simulink model "fftwbr_core"  (Simulink 7.9)
settings: Solver=ode45  StartTime=0.0  StopTime=10.0  RelTol=1e-3
libraries: xbsIndex_r4 x15792, casper_library_misc x464, casper_library_delays x57, xbsReplacements_r4 x8, casper_library_ffts_twiddle x4
(part 135 of 233)

== system fftwbr_core/fft_wideband_real1/fft_direct/butterfly4_4/twiddle_general_dsp48e/coeff_gen ==
  rst : Inport
  Counter : xbsIndex_r4/Counter  cnt_type=Free Running  cnt_to=Inf  operation=Up  start_count=0  cnt_by_val=1  arith_type=Unsigned  n_bits=11  bin_pt=0  load_pin=off  rst=on  en=off  explicit_period=on  period=1  dbl_ovrd=off  use_behavioral_HDL=off  implementation=Fabric  block_type=counter
  ROM : xbsIndex_r4/ROM  depth=2048  initVector="[0.9238739013671875                     0.9238128662109375                      0.923736572265625                     0.9236602783203125                         0.923583984375                     0.9235076904296875                      0.923431396484375                      0.923370361328125                     0.9232940673828125                        0.9232177734375                     0.9231414794921875                      0.923065185546875                      0.923004150390625                     0.9229278564453125                           0.9228515625                     0.9227752685546875                      0.922698974609375                     0.9226226806640625                       0.92254638671875                        0.9224853515625                     0.9224090576171875                      0.922332763671875                     0.9222564697265625                       0.92218017578125                     0.9221038818359375                      0.922027587890625                      0.921966552734375                     0.9218902587890625                       0.92181396484375                     0.9217376708984375                      0.921661376953125                     0.9215850830078125                        0.9215087890625                     0.9214324951171875                     0.9213714599609375                      0.921295166015625                     0.9212188720703125                         0.921142578125                     0.9210662841796875                      0.920989990234375                     0.9209136962890625                       0.92083740234375                     0.9207611083984375                     0.9207000732421875                      0.920623779296875                     0.9205474853515625                       0.92047119140625                     0.9203948974609375                      0.920318603515625                     0.9202423095703125                         0.920166015625                     0.9200897216796875                      0.920013427734375                     0.9199371337890625                       0.91986083984375                        0.9197998046875                     0.9197235107421875                      0.919647216796875                     0.9195709228515625                       0.91949462890625                     0.9194183349609375                      0.919342041015625                     0.9192657470703125                         0.919189453125                     0.9191131591796875                      0.919036865234375                     0.9189605712890625                       0.91888427734375                     0.9188079833984375                      0.918731689453125                     0.9186553955078125                        0.9185791015625                     0.9185028076171875                      0.918426513671875                     0.9183502197265625                       0.91827392578125                     0.9181976318359375                      0.918121337890625                      0.918060302734375                     0.9179840087890625                       0.91790771484375                     0.9178314208984375                      0.917755126953125                     0.9176788330078125                        0.9176025390625                     0.9175262451171875                      0.917449951171875                     0.9173736572265625                       0.91729736328125                     0.9172210693359375                      0.917144775390625                     0.9170684814453125                           0.9169921875                     0.9169158935546875                      0.916839599609375                         0.916748046875                     0.9166717529296875                      0.916595458984375                     0.9165191650390625                       0.91644287109375                     0.9163665771484375                      0.916290283203125                     0.9162139892578125 ...(+75853 chars)]"  distributed_mem=Block RAM  rst=off  init_reg=0  en=off  latency=2  gui_display_data_type=Fixed-point  arith_type="Signed  (2's comp)"  n_bits=18  bin_pt=16  preci_type=Single  exp_width=8  frac_width=24  dbl_ovrd=off  optimize=Area  use_rpm=on  block_type=sprom
  ROM1 : xbsIndex_r4/ROM  depth=2048  initVector="[-0.3826904296875                    -0.3828582763671875                    -0.3830413818359375                     -0.383209228515625                     -0.383392333984375                     -0.383575439453125                    -0.3837432861328125                    -0.3839263916015625                      -0.38409423828125                         -0.38427734375                      -0.38446044921875                    -0.3846282958984375                    -0.3848114013671875                     -0.384979248046875                     -0.385162353515625                     -0.385345458984375                    -0.3855133056640625                    -0.3856964111328125                       -0.3858642578125                      -0.38604736328125                         -0.38623046875                    -0.3863983154296875                    -0.3865814208984375                     -0.386749267578125                     -0.386932373046875                    -0.3871002197265625                    -0.3872833251953125                    -0.3874664306640625                      -0.38763427734375                       -0.3878173828125                    -0.3879852294921875                    -0.3881683349609375                    -0.3883514404296875                     -0.388519287109375                     -0.388702392578125                    -0.3888702392578125                    -0.3890533447265625                      -0.38922119140625                        -0.389404296875                      -0.38958740234375                    -0.3897552490234375                    -0.3899383544921875                     -0.390106201171875                     -0.390289306640625                    -0.3904571533203125                    -0.3906402587890625                    -0.3908233642578125                       -0.3909912109375                      -0.39117431640625                    -0.3913421630859375                    -0.3915252685546875                     -0.391693115234375                     -0.391876220703125                     -0.392059326171875                    -0.3922271728515625                    -0.3924102783203125                           -0.392578125                      -0.39276123046875                    -0.3929290771484375                    -0.3931121826171875                     -0.393280029296875                     -0.393463134765625                     -0.393646240234375                    -0.3938140869140625                    -0.3939971923828125                       -0.3941650390625                      -0.39434814453125                    -0.3945159912109375                    -0.3946990966796875                     -0.394866943359375                     -0.395050048828125                    -0.3952178955078125                    -0.3954010009765625                    -0.3955841064453125                        -0.395751953125                      -0.39593505859375                    -0.3961029052734375                    -0.3962860107421875                     -0.396453857421875                     -0.396636962890625                    -0.3968048095703125                    -0.3969879150390625                      -0.39715576171875                       -0.3973388671875                    -0.3975067138671875                    -0.3976898193359375                    -0.3978729248046875                     -0.398040771484375                     -0.398223876953125                    -0.3983917236328125                    -0.3985748291015625                      -0.39874267578125                         -0.39892578125                    -0.3990936279296875                    -0.3992767333984375                     -0.399444580078125                     -0.399627685546875                    -0.3997955322265625                    -0.3999786376953125                        -0.400146484375                      -0.40032958984375                    -0.4004974365234375                    -0.4006805419921875 ...(+75851 chars)]"  distributed_mem=Block RAM  rst=off  init_reg=0  en=off  latency=2  gui_display_data_type=Fixed-point  arith_type="Signed  (2's comp)"  n_bits=18  bin_pt=16  preci_type=Single  exp_width=8  frac_width=24  dbl_ovrd=off  optimize=Area  use_rpm=on  block_type=sprom
  Slice : xbsIndex_r4/Slice  nbits=11  boolean_output=off  mode=Upper Bit Location + Width  bit1=0  base1=MSB of Input  bit0=0  base0=LSB of Input  dbl_ovrd=off  block_type=slice
  ri_to_c : casper_library_misc/ri_to_c
  w : Outport
  wires:
  ri_to_c/1 -> w/1
  rst/1 -> Counter/1
  Counter/1 -> Slice/1
  Slice/1 -> ROM/1, ROM1/1
  ROM/1 -> ri_to_c/1
  ROM1/1 -> ri_to_c/2
